simulink model "rpoco8_testbench_v11"  (Simulink 7.4)
settings: Solver=ode45  StartTime=0.0  StopTime=10.0  RelTol=1e-3
undefined symbols (not defined in the file): quant
libraries: xbsIndex_r4 x1012, xps_library x76, casper_library_misc x55, casper_library_multipliers x19, casper_library_delays x9, casper_library_sources x4, simulink x1
(part 6 of 11)

== system rpoco8_testbench_v11/xengine8_muxed/be ==
  a+bi : Inport  OutDataType=sfix(16)  OutScaling=2^0
  c+di : Inport  Port=2  OutDataType=sfix(16)  OutScaling=2^0
  new_acc : Inport  Port=3  OutDataType=sfix(16)  OutScaling=2^0
  Convert{,1..3} (4 alike) : xbsIndex_r4/Convert  arith_type="Signed  (2's comp)"  n_bits=16  bin_pt=15  quantization="Round  (unbiased: Even Values)"  overflow=Wrap  latency=0  dbl_ovrd=off  pipeline=off  block_type=convert
      settings: block_version=11.4
  Counter2 : xbsIndex_r4/Counter  cnt_type=Free Running  cnt_to=Inf  operation=Up  start_count=0  cnt_by_val=1  arith_type=Unsigned  n_bits=max(nchan+1,11)  bin_pt=0  load_pin=off  rst=on  en=on  explicit_period=on  period=1  dbl_ovrd=off  use_behavioral_HDL=off  implementation=Fabric  use_rpm=off  block_type=counter
  Delay1 : xbsIndex_r4/Delay  en=off  latency=mult_latency + add_latency  dbl_ovrd=off  reg_retiming=off  block_type=delay
  c_to_ri : casper_library_misc/c_to_ri  SystemSampleTime=-1  n_bits=n_bits  bin_pt=n_bits-1
  c_to_ri1 : casper_library_misc/c_to_ri  SystemSampleTime=-1  n_bits=n_bits  bin_pt=n_bits-1
  cmult_4bit_em* : casper_library_multipliers/cmult_4bit_em*  SystemSampleTime=-1  mult_latency=mult_latency  add_latency=add_latency
  imag : xps_library/Shared BRAM  Tag=xps:bram  SystemSampleTime=-1  arith_type="Signed  (2's comp)"  addr_width=max(nchan+1,11)  data_width=32  data_bin_pt=min(2*(n_bits-1), 30)  init_vals=0  sample_rate=1
      link override convert_din: arith_type="Signed  (2's comp)"
      link override i_poco8_128ch_v004_mod_xengine8_muxed_ab_imag_data_out: arith_type="Signed  (2's comp)"
      link override i_poco8_256ch_v000_xengine8_muxed_ab_imag_data_out: arith_type="Signed  (2's comp)"
      link override i_poco8_256ch_v004_mod_xengine8_muxed_ab_imag_data_out: arith_type="Signed  (2's comp)"
      link override i_poco8_256ch_v004_xengine8_muxed_ab_imag_data_out: arith_type="Signed  (2's comp)"
      link override i_poco8_32ch_v001_xengine8_muxed_ab_imag_data_out: arith_type="Signed  (2's comp)"
      link override i_poco8_32ch_v002_xengine8_muxed_ab_imag_data_out: arith_type="Signed  (2's comp)"
      link override i_poco8_32ch_v003_xengine8_muxed_ab_imag_data_out: arith_type="Signed  (2's comp)"
      link override i_poco8_test_xengine8_muxed_ab_imag_data_out: arith_type="Signed  (2's comp)"
      link override mem/sim_data_in: arith_type="Signed  (2's comp)"
      link override poco4_quad_200_4k_01_xengine4_x_cross_sl1_imag_data_out: arith_type="Signed  (2's comp)"
      link override r_poco8_4k_10_200_xengine8_x_cross_sl1_imag_data_out: arith_type="Signed  (2's comp)"
      link override r_poco8_4k_10_200a_xengine8_ab_imag_data_out: arith_type="Signed  (2's comp)"
  real : xps_library/Shared BRAM  Tag=xps:bram  SystemSampleTime=-1  arith_type="Signed  (2's comp)"  addr_width=max(nchan+1,11)  data_width=32  data_bin_pt=min(2*(n_bits-1), 30)  init_vals=0  sample_rate=1
      link override convert_din: arith_type="Signed  (2's comp)"
      link override i_poco8_128ch_v004_mod_xengine8_muxed_ab_real_data_out: arith_type="Signed  (2's comp)"
      link override i_poco8_256ch_v000_xengine8_muxed_ab_real_data_out: arith_type="Signed  (2's comp)"
      link override i_poco8_256ch_v004_mod_xengine8_muxed_ab_real_data_out: arith_type="Signed  (2's comp)"
      link override i_poco8_256ch_v004_xengine8_muxed_ab_real_data_out: arith_type="Signed  (2's comp)"
      link override i_poco8_32ch_v001_xengine8_muxed_ab_real_data_out: arith_type="Signed  (2's comp)"
      link override i_poco8_32ch_v002_xengine8_muxed_ab_real_data_out: arith_type="Signed  (2's comp)"
      link override i_poco8_32ch_v003_xengine8_muxed_ab_real_data_out: arith_type="Signed  (2's comp)"
      link override i_poco8_test_xengine8_muxed_ab_real_data_out: arith_type="Signed  (2's comp)"
      link override mem/sim_data_in: arith_type="Signed  (2's comp)"
      link override poco4_quad_200_4k_01_xengine4_x_cross_sl1_real_data_out: arith_type="Signed  (2's comp)"
      link override r_poco8_4k_10_200_xengine8_x_cross_sl1_real_data_out: arith_type="Signed  (2's comp)"
      link override r_poco8_4k_10_200a_xengine8_ab_real_data_out: arith_type="Signed  (2's comp)"
  simple_bram_vacc : Subsystem  AncestorBlock=casper_library/Accumulators/simple_bram_vacc  mask(simple_bram_vacc){vec_len=2048, arith_type=Signed, n_bits=32, bin_pt=30}
      mask prompts: vec_len "Vector length:"; arith_type "Output type:"; n_bits "Bit width (output):"; bin_pt "Binary point (output):"
      mask help: This simple vector accumulator is based on a shift register. It outputs its previous accumulated vector after a new_acc pulse is received.
      mask init:
      | simple_bram_vacc_init(gcb, ...
      |     'vec_len', vec_len, ...
      |     'arith_type', arith_type, ...
      |     'n_bits', n_bits, ...
      |     'bin_pt', bin_pt);
      mask StyleString: edit,popup(Signed|Unsigned),edit,edit
  simple_bram_vacc1 : Subsystem  AncestorBlock=casper_library/Accumulators/simple_bram_vacc  mask(simple_bram_vacc){vec_len=2048, arith_type=Signed, n_bits=32, bin_pt=30}
      mask prompts: vec_len "Vector length:"; arith_type "Output type:"; n_bits "Bit width (output):"; bin_pt "Binary point (output):"
      mask help: This simple vector accumulator is based on a shift register. It outputs its previous accumulated vector after a new_acc pulse is received.
      mask init:
      | simple_bram_vacc_init(gcb, ...
      |     'vec_len', vec_len, ...
      |     'arith_type', arith_type, ...
      |     'n_bits', n_bits, ...
      |     'bin_pt', bin_pt);
      mask StyleString: edit,popup(Signed|Unsigned),edit,edit
  wires:
  cmult_4bit_em*/2 -> simple_bram_vacc1/2(din)
  cmult_4bit_em*/1 -> simple_bram_vacc/2(din)
  simple_bram_vacc1/2(valid) -> imag/3
  simple_bram_vacc1/1(dout) -> imag/2
  Delay1/1 -> Counter2/1, simple_bram_vacc/1(new_acc), simple_bram_vacc1/1(new_acc)
  simple_bram_vacc/1(dout) -> real/2
  Counter2/1 -> imag/1, real/1
  simple_bram_vacc/2(valid) -> real/3, Counter2/2
  new_acc/1 -> Delay1/1
  c+di/1 -> c_to_ri1/1
  a+bi/1 -> c_to_ri/1
  c_to_ri1/2 -> Convert3/1
  c_to_ri1/1 -> Convert2/1
  c_to_ri/2 -> Convert1/1
  c_to_ri/1 -> Convert/1
  Convert/1 -> cmult_4bit_em*/1
  Convert1/1 -> cmult_4bit_em*/2
  Convert2/1 -> cmult_4bit_em*/3
  Convert3/1 -> cmult_4bit_em*/4

== system rpoco8_testbench_v11/xengine8_muxed/bf ==
  a+bi : Inport  OutDataType=sfix(16)  OutScaling=2^0
  c+di : Inport  Port=2  OutDataType=sfix(16)  OutScaling=2^0
  new_acc : Inport  Port=3  OutDataType=sfix(16)  OutScaling=2^0
  Convert{,1..3} (4 alike) : xbsIndex_r4/Convert  arith_type="Signed  (2's comp)"  n_bits=16  bin_pt=15  quantization="Round  (unbiased: Even Values)"  overflow=Wrap  latency=0  dbl_ovrd=off  pipeline=off  block_type=convert
      settings: block_version=11.4
  Counter2 : xbsIndex_r4/Counter  cnt_type=Free Running  cnt_to=Inf  operation=Up  start_count=0  cnt_by_val=1  arith_type=Unsigned  n_bits=max(nchan+1,11)  bin_pt=0  load_pin=off  rst=on  en=on  explicit_period=on  period=1  dbl_ovrd=off  use_behavioral_HDL=off  implementation=Fabric  use_rpm=off  block_type=counter
  Delay1 : xbsIndex_r4/Delay  en=off  latency=mult_latency + add_latency  dbl_ovrd=off  reg_retiming=off  block_type=delay
  c_to_ri : casper_library_misc/c_to_ri  SystemSampleTime=-1  n_bits=n_bits  bin_pt=n_bits-1
  c_to_ri1 : casper_library_misc/c_to_ri  SystemSampleTime=-1  n_bits=n_bits  bin_pt=n_bits-1
  cmult_4bit_em* : casper_library_multipliers/cmult_4bit_em*  SystemSampleTime=-1  mult_latency=mult_latency  add_latency=add_latency
  imag : xps_library/Shared BRAM  Tag=xps:bram  SystemSampleTime=-1  arith_type="Signed  (2's comp)"  addr_width=max(nchan+1,11)  data_width=32  data_bin_pt=min(2*(n_bits-1), 30)  init_vals=0  sample_rate=1
      link override convert_din: arith_type="Signed  (2's comp)"
      link override i_poco8_128ch_v004_mod_xengine8_muxed_ab_imag_data_out: arith_type="Signed  (2's comp)"
      link override i_poco8_256ch_v000_xengine8_muxed_ab_imag_data_out: arith_type="Signed  (2's comp)"
      link override i_poco8_256ch_v004_mod_xengine8_muxed_ab_imag_data_out: arith_type="Signed  (2's comp)"
      link override i_poco8_256ch_v004_xengine8_muxed_ab_imag_data_out: arith_type="Signed  (2's comp)"
      link override i_poco8_32ch_v001_xengine8_muxed_ab_imag_data_out: arith_type="Signed  (2's comp)"
      link override i_poco8_32ch_v002_xengine8_muxed_ab_imag_data_out: arith_type="Signed  (2's comp)"
      link override i_poco8_32ch_v003_xengine8_muxed_ab_imag_data_out: arith_type="Signed  (2's comp)"
      link override i_poco8_test_xengine8_muxed_ab_imag_data_out: arith_type="Signed  (2's comp)"
      link override mem/sim_data_in: arith_type="Signed  (2's comp)"
      link override poco4_quad_200_4k_01_xengine4_x_cross_sl1_imag_data_out: arith_type="Signed  (2's comp)"
      link override r_poco8_4k_10_200_xengine8_x_cross_sl1_imag_data_out: arith_type="Signed  (2's comp)"
      link override r_poco8_4k_10_200a_xengine8_ab_imag_data_out: arith_type="Signed  (2's comp)"
  real : xps_library/Shared BRAM  Tag=xps:bram  SystemSampleTime=-1  arith_type="Signed  (2's comp)"  addr_width=max(nchan+1,11)  data_width=32  data_bin_pt=min(2*(n_bits-1), 30)  init_vals=0  sample_rate=1
      link override convert_din: arith_type="Signed  (2's comp)"
      link override i_poco8_128ch_v004_mod_xengine8_muxed_ab_real_data_out: arith_type="Signed  (2's comp)"
      link override i_poco8_256ch_v000_xengine8_muxed_ab_real_data_out: arith_type="Signed  (2's comp)"
      link override i_poco8_256ch_v004_mod_xengine8_muxed_ab_real_data_out: arith_type="Signed  (2's comp)"
      link override i_poco8_256ch_v004_xengine8_muxed_ab_real_data_out: arith_type="Signed  (2's comp)"
      link override i_poco8_32ch_v001_xengine8_muxed_ab_real_data_out: arith_type="Signed  (2's comp)"
      link override i_poco8_32ch_v002_xengine8_muxed_ab_real_data_out: arith_type="Signed  (2's comp)"
      link override i_poco8_32ch_v003_xengine8_muxed_ab_real_data_out: arith_type="Signed  (2's comp)"
      link override i_poco8_test_xengine8_muxed_ab_real_data_out: arith_type="Signed  (2's comp)"
      link override mem/sim_data_in: arith_type="Signed  (2's comp)"
      link override poco4_quad_200_4k_01_xengine4_x_cross_sl1_real_data_out: arith_type="Signed  (2's comp)"
      link override r_poco8_4k_10_200_xengine8_x_cross_sl1_real_data_out: arith_type="Signed  (2's comp)"
      link override r_poco8_4k_10_200a_xengine8_ab_real_data_out: arith_type="Signed  (2's comp)"
  simple_bram_vacc : Subsystem  AncestorBlock=casper_library/Accumulators/simple_bram_vacc  mask(simple_bram_vacc){vec_len=2048, arith_type=Signed, n_bits=32, bin_pt=30}
      mask prompts: vec_len "Vector length:"; arith_type "Output type:"; n_bits "Bit width (output):"; bin_pt "Binary point (output):"
      mask help: This simple vector accumulator is based on a shift register. It outputs its previous accumulated vector after a new_acc pulse is received.
      mask init:
      | simple_bram_vacc_init(gcb, ...
      |     'vec_len', vec_len, ...
      |     'arith_type', arith_type, ...
      |     'n_bits', n_bits, ...
      |     'bin_pt', bin_pt);
      mask StyleString: edit,popup(Signed|Unsigned),edit,edit
  simple_bram_vacc1 : Subsystem  AncestorBlock=casper_library/Accumulators/simple_bram_vacc  mask(simple_bram_vacc){vec_len=2048, arith_type=Signed, n_bits=32, bin_pt=30}
      mask prompts: vec_len "Vector length:"; arith_type "Output type:"; n_bits "Bit width (output):"; bin_pt "Binary point (output):"
      mask help: This simple vector accumulator is based on a shift register. It outputs its previous accumulated vector after a new_acc pulse is received.
      mask init:
      | simple_bram_vacc_init(gcb, ...
      |     'vec_len', vec_len, ...
      |     'arith_type', arith_type, ...
      |     'n_bits', n_bits, ...
      |     'bin_pt', bin_pt);
      mask StyleString: edit,popup(Signed|Unsigned),edit,edit
  wires:
  cmult_4bit_em*/2 -> simple_bram_vacc1/2(din)
  cmult_4bit_em*/1 -> simple_bram_vacc/2(din)
  simple_bram_vacc1/2(valid) -> imag/3
  simple_bram_vacc1/1(dout) -> imag/2
  Delay1/1 -> Counter2/1, simple_bram_vacc/1(new_acc), simple_bram_vacc1/1(new_acc)
  simple_bram_vacc/1(dout) -> real/2
  Counter2/1 -> imag/1, real/1
  simple_bram_vacc/2(valid) -> real/3, Counter2/2
  new_acc/1 -> Delay1/1
  c+di/1 -> c_to_ri1/1
  a+bi/1 -> c_to_ri/1
  c_to_ri1/2 -> Convert3/1
  c_to_ri1/1 -> Convert2/1
  c_to_ri/2 -> Convert1/1
  c_to_ri/1 -> Convert/1
  Convert/1 -> cmult_4bit_em*/1
  Convert1/1 -> cmult_4bit_em*/2
  Convert2/1 -> cmult_4bit_em*/3
  Convert3/1 -> cmult_4bit_em*/4

== system rpoco8_testbench_v11/xengine8_muxed/cc ==
  a+bi : Inport  OutDataType=sfix(16)  OutScaling=2^0
  c+di : Inport  Port=2  OutDataType=sfix(16)  OutScaling=2^0
  new_acc : Inport  Port=3  OutDataType=sfix(16)  OutScaling=2^0
  Convert{,1..3} (4 alike) : xbsIndex_r4/Convert  arith_type="Signed  (2's comp)"  n_bits=16  bin_pt=15  quantization="Round  (unbiased: Even Values)"  overflow=Wrap  latency=0  dbl_ovrd=off  pipeline=off  block_type=convert
      settings: block_version=11.4
  Counter2 : xbsIndex_r4/Counter  cnt_type=Free Running  cnt_to=Inf  operation=Up  start_count=0  cnt_by_val=1  arith_type=Unsigned  n_bits=max(nchan+1,11)  bin_pt=0  load_pin=off  rst=on  en=on  explicit_period=on  period=1  dbl_ovrd=off  use_behavioral_HDL=off  implementation=Fabric  use_rpm=off  block_type=counter
  Delay1 : xbsIndex_r4/Delay  en=off  latency=mult_latency + add_latency  dbl_ovrd=off  reg_retiming=off  block_type=delay
  c_to_ri : casper_library_misc/c_to_ri  SystemSampleTime=-1  n_bits=n_bits  bin_pt=n_bits-1
  c_to_ri1 : casper_library_misc/c_to_ri  SystemSampleTime=-1  n_bits=n_bits  bin_pt=n_bits-1
  cmult_4bit_em* : casper_library_multipliers/cmult_4bit_em*  SystemSampleTime=-1  mult_latency=mult_latency  add_latency=add_latency
  real : xps_library/Shared BRAM  Tag=xps:bram  SystemSampleTime=-1  arith_type="Signed  (2's comp)"  addr_width=max(nchan+1,11)  data_width=32  data_bin_pt=min(2*(n_bits-1), 30)  init_vals=0  sample_rate=1
      link override convert_din: arith_type="Signed  (2's comp)"
      link override i_poco8_128ch_v004_mod_xengine8_muxed_aa_real_data_out: arith_type="Signed  (2's comp)"
      link override i_poco8_256ch_v000_xengine8_muxed_aa_real_data_out: arith_type="Signed  (2's comp)"
      link override i_poco8_256ch_v004_mod_xengine8_muxed_aa_real_data_out: arith_type="Signed  (2's comp)"
      link override i_poco8_256ch_v004_xengine8_muxed_aa_real_data_out: arith_type="Signed  (2's comp)"
      link override i_poco8_32ch_v001_xengine8_muxed_aa_real_data_out: arith_type="Signed  (2's comp)"
      link override i_poco8_32ch_v002_xengine8_muxed_aa_real_data_out: arith_type="Signed  (2's comp)"
      link override i_poco8_32ch_v003_xengine8_muxed_aa_real_data_out: arith_type="Signed  (2's comp)"
      link override i_poco8_test_xengine8_muxed_aa_real_data_out: arith_type="Signed  (2's comp)"
      link override mem/sim_data_in: arith_type="Signed  (2's comp)"
      link override poco4_quad_200_4k_01_xengine4_x_auto_sl_real_data_out: arith_type="Signed  (2's comp)"
      link override r_poco8_4k_10_200_xengine8_x_auto_sl_real_data_out: arith_type="Signed  (2's comp)"
      link override r_poco8_4k_10_200a_xengine8_aa_real_data_out: arith_type="Signed  (2's comp)"
  simple_bram_vacc : Subsystem  AncestorBlock=casper_library/Accumulators/simple_bram_vacc  mask(simple_bram_vacc){vec_len=2048, arith_type=Signed, n_bits=32, bin_pt=30}
      mask prompts: vec_len "Vector length:"; arith_type "Output type:"; n_bits "Bit width (output):"; bin_pt "Binary point (output):"
      mask help: This simple vector accumulator is based on a shift register. It outputs its previous accumulated vector after a new_acc pulse is received.
      mask init:
      | simple_bram_vacc_init(gcb, ...
      |     'vec_len', vec_len, ...
      |     'arith_type', arith_type, ...
      |     'n_bits', n_bits, ...
      |     'bin_pt', bin_pt);
      mask StyleString: edit,popup(Signed|Unsigned),edit,edit
  wires:
  cmult_4bit_em*/1 -> simple_bram_vacc/2(din)
  new_acc/1 -> Delay1/1
  c+di/1 -> c_to_ri1/1
  a+bi/1 -> c_to_ri/1
  simple_bram_vacc/2(valid) -> Counter2/2, real/3
  Counter2/1 -> real/1
  simple_bram_vacc/1(dout) -> real/2
  Delay1/1 -> Counter2/1, simple_bram_vacc/1(new_acc)
  c_to_ri1/2 -> Convert3/1
  c_to_ri1/1 -> Convert2/1
  c_to_ri/2 -> Convert1/1
  c_to_ri/1 -> Convert/1
  Convert/1 -> cmult_4bit_em*/1
  Convert1/1 -> cmult_4bit_em*/2
  Convert2/1 -> cmult_4bit_em*/3
  Convert3/1 -> cmult_4bit_em*/4

== system rpoco8_testbench_v11/xengine8_muxed/cd ==
  a+bi : Inport  OutDataType=sfix(16)  OutScaling=2^0
  c+di : Inport  Port=2  OutDataType=sfix(16)  OutScaling=2^0
  new_acc : Inport  Port=3  OutDataType=sfix(16)  OutScaling=2^0
  Convert{,1..3} (4 alike) : xbsIndex_r4/Convert  arith_type="Signed  (2's comp)"  n_bits=16  bin_pt=15  quantization="Round  (unbiased: Even Values)"  overflow=Wrap  latency=0  dbl_ovrd=off  pipeline=off  block_type=convert
      settings: block_version=11.4
  Counter2 : xbsIndex_r4/Counter  cnt_type=Free Running  cnt_to=Inf  operation=Up  start_count=0  cnt_by_val=1  arith_type=Unsigned  n_bits=max(nchan+1,11)  bin_pt=0  load_pin=off  rst=on  en=on  explicit_period=on  period=1  dbl_ovrd=off  use_behavioral_HDL=off  implementation=Fabric  use_rpm=off  block_type=counter
  Delay1 : xbsIndex_r4/Delay  en=off  latency=mult_latency + add_latency  dbl_ovrd=off  reg_retiming=off  block_type=delay
  c_to_ri : casper_library_misc/c_to_ri  SystemSampleTime=-1  n_bits=n_bits  bin_pt=n_bits-1
  c_to_ri1 : casper_library_misc/c_to_ri  SystemSampleTime=-1  n_bits=n_bits  bin_pt=n_bits-1
  cmult_4bit_em* : casper_library_multipliers/cmult_4bit_em*  SystemSampleTime=-1  mult_latency=mult_latency  add_latency=add_latency
  imag : xps_library/Shared BRAM  Tag=xps:bram  SystemSampleTime=-1  arith_type="Signed  (2's comp)"  addr_width=max(nchan+1,11)  data_width=32  data_bin_pt=min(2*(n_bits-1), 30)  init_vals=0  sample_rate=1
      link override convert_din: arith_type="Signed  (2's comp)"
      link override i_poco8_128ch_v004_mod_xengine8_muxed_ab_imag_data_out: arith_type="Signed  (2's comp)"
      link override i_poco8_256ch_v000_xengine8_muxed_ab_imag_data_out: arith_type="Signed  (2's comp)"
      link override i_poco8_256ch_v004_mod_xengine8_muxed_ab_imag_data_out: arith_type="Signed  (2's comp)"
      link override i_poco8_256ch_v004_xengine8_muxed_ab_imag_data_out: arith_type="Signed  (2's comp)"
      link override i_poco8_32ch_v001_xengine8_muxed_ab_imag_data_out: arith_type="Signed  (2's comp)"
      link override i_poco8_32ch_v002_xengine8_muxed_ab_imag_data_out: arith_type="Signed  (2's comp)"
      link override i_poco8_32ch_v003_xengine8_muxed_ab_imag_data_out: arith_type="Signed  (2's comp)"
      link override i_poco8_test_xengine8_muxed_ab_imag_data_out: arith_type="Signed  (2's comp)"
      link override mem/sim_data_in: arith_type="Signed  (2's comp)"
      link override poco4_quad_200_4k_01_xengine4_x_cross_sl1_imag_data_out: arith_type="Signed  (2's comp)"
      link override r_poco8_4k_10_200_xengine8_x_cross_sl1_imag_data_out: arith_type="Signed  (2's comp)"
      link override r_poco8_4k_10_200a_xengine8_ab_imag_data_out: arith_type="Signed  (2's comp)"
  real : xps_library/Shared BRAM  Tag=xps:bram  SystemSampleTime=-1  arith_type="Signed  (2's comp)"  addr_width=max(nchan+1,11)  data_width=32  data_bin_pt=min(2*(n_bits-1), 30)  init_vals=0  sample_rate=1
      link override convert_din: arith_type="Signed  (2's comp)"
      link override i_poco8_128ch_v004_mod_xengine8_muxed_ab_real_data_out: arith_type="Signed  (2's comp)"
      link override i_poco8_256ch_v000_xengine8_muxed_ab_real_data_out: arith_type="Signed  (2's comp)"
      link override i_poco8_256ch_v004_mod_xengine8_muxed_ab_real_data_out: arith_type="Signed  (2's comp)"
      link override i_poco8_256ch_v004_xengine8_muxed_ab_real_data_out: arith_type="Signed  (2's comp)"
      link override i_poco8_32ch_v001_xengine8_muxed_ab_real_data_out: arith_type="Signed  (2's comp)"
      link override i_poco8_32ch_v002_xengine8_muxed_ab_real_data_out: arith_type="Signed  (2's comp)"
      link override i_poco8_32ch_v003_xengine8_muxed_ab_real_data_out: arith_type="Signed  (2's comp)"
      link override i_poco8_test_xengine8_muxed_ab_real_data_out: arith_type="Signed  (2's comp)"
      link override mem/sim_data_in: arith_type="Signed  (2's comp)"
      link override poco4_quad_200_4k_01_xengine4_x_cross_sl1_real_data_out: arith_type="Signed  (2's comp)"
      link override r_poco8_4k_10_200_xengine8_x_cross_sl1_real_data_out: arith_type="Signed  (2's comp)"
      link override r_poco8_4k_10_200a_xengine8_ab_real_data_out: arith_type="Signed  (2's comp)"
  simple_bram_vacc : Subsystem  AncestorBlock=casper_library/Accumulators/simple_bram_vacc  mask(simple_bram_vacc){vec_len=2048, arith_type=Signed, n_bits=32, bin_pt=30}
      mask prompts: vec_len "Vector length:"; arith_type "Output type:"; n_bits "Bit width (output):"; bin_pt "Binary point (output):"
      mask help: This simple vector accumulator is based on a shift register. It outputs its previous accumulated vector after a new_acc pulse is received.
      mask init:
      | simple_bram_vacc_init(gcb, ...
      |     'vec_len', vec_len, ...
      |     'arith_type', arith_type, ...
      |     'n_bits', n_bits, ...
      |     'bin_pt', bin_pt);
      mask StyleString: edit,popup(Signed|Unsigned),edit,edit
  simple_bram_vacc1 : Subsystem  AncestorBlock=casper_library/Accumulators/simple_bram_vacc  mask(simple_bram_vacc){vec_len=2048, arith_type=Signed, n_bits=32, bin_pt=30}
      mask prompts: vec_len "Vector length:"; arith_type "Output type:"; n_bits "Bit width (output):"; bin_pt "Binary point (output):"
      mask help: This simple vector accumulator is based on a shift register. It outputs its previous accumulated vector after a new_acc pulse is received.
      mask init:
      | simple_bram_vacc_init(gcb, ...
      |     'vec_len', vec_len, ...
      |     'arith_type', arith_type, ...
      |     'n_bits', n_bits, ...
      |     'bin_pt', bin_pt);
      mask StyleString: edit,popup(Signed|Unsigned),edit,edit
  wires:
  cmult_4bit_em*/2 -> simple_bram_vacc1/2(din)
  cmult_4bit_em*/1 -> simple_bram_vacc/2(din)
  simple_bram_vacc1/2(valid) -> imag/3
  simple_bram_vacc1/1(dout) -> imag/2
  Delay1/1 -> Counter2/1, simple_bram_vacc/1(new_acc), simple_bram_vacc1/1(new_acc)
  simple_bram_vacc/1(dout) -> real/2
  Counter2/1 -> imag/1, real/1
  simple_bram_vacc/2(valid) -> real/3, Counter2/2
  new_acc/1 -> Delay1/1
  c+di/1 -> c_to_ri1/1
  a+bi/1 -> c_to_ri/1
  c_to_ri1/2 -> Convert3/1
  c_to_ri1/1 -> Convert2/1
  c_to_ri/2 -> Convert1/1
  c_to_ri/1 -> Convert/1
  Convert/1 -> cmult_4bit_em*/1
  Convert1/1 -> cmult_4bit_em*/2
  Convert2/1 -> cmult_4bit_em*/3
  Convert3/1 -> cmult_4bit_em*/4

== system rpoco8_testbench_v11/xengine8_muxed/cg ==
  a+bi : Inport  OutDataType=sfix(16)  OutScaling=2^0
  c+di : Inport  Port=2  OutDataType=sfix(16)  OutScaling=2^0
  new_acc : Inport  Port=3  OutDataType=sfix(16)  OutScaling=2^0
  Convert{,1..3} (4 alike) : xbsIndex_r4/Convert  arith_type="Signed  (2's comp)"  n_bits=16  bin_pt=15  quantization="Round  (unbiased: Even Values)"  overflow=Wrap  latency=0  dbl_ovrd=off  pipeline=off  block_type=convert
      settings: block_version=11.4
  Counter2 : xbsIndex_r4/Counter  cnt_type=Free Running  cnt_to=Inf  operation=Up  start_count=0  cnt_by_val=1  arith_type=Unsigned  n_bits=max(nchan+1,11)  bin_pt=0  load_pin=off  rst=on  en=on  explicit_period=on  period=1  dbl_ovrd=off  use_behavioral_HDL=off  implementation=Fabric  use_rpm=off  block_type=counter
  Delay1 : xbsIndex_r4/Delay  en=off  latency=mult_latency + add_latency  dbl_ovrd=off  reg_retiming=off  block_type=delay
  c_to_ri : casper_library_misc/c_to_ri  SystemSampleTime=-1  n_bits=n_bits  bin_pt=n_bits-1
  c_to_ri1 : casper_library_misc/c_to_ri  SystemSampleTime=-1  n_bits=n_bits  bin_pt=n_bits-1
  cmult_4bit_em* : casper_library_multipliers/cmult_4bit_em*  SystemSampleTime=-1  mult_latency=mult_latency  add_latency=add_latency
  imag : xps_library/Shared BRAM  Tag=xps:bram  SystemSampleTime=-1  arith_type="Signed  (2's comp)"  addr_width=max(nchan+1,11)  data_width=32  data_bin_pt=min(2*(n_bits-1), 30)  init_vals=0  sample_rate=1
      link override convert_din: arith_type="Signed  (2's comp)"
      link override i_poco8_128ch_v004_mod_xengine8_muxed_ab_imag_data_out: arith_type="Signed  (2's comp)"
      link override i_poco8_256ch_v000_xengine8_muxed_ab_imag_data_out: arith_type="Signed  (2's comp)"
      link override i_poco8_256ch_v004_mod_xengine8_muxed_ab_imag_data_out: arith_type="Signed  (2's comp)"
      link override i_poco8_256ch_v004_xengine8_muxed_ab_imag_data_out: arith_type="Signed  (2's comp)"
      link override i_poco8_32ch_v001_xengine8_muxed_ab_imag_data_out: arith_type="Signed  (2's comp)"
      link override i_poco8_32ch_v002_xengine8_muxed_ab_imag_data_out: arith_type="Signed  (2's comp)"
      link override i_poco8_32ch_v003_xengine8_muxed_ab_imag_data_out: arith_type="Signed  (2's comp)"
      link override i_poco8_test_xengine8_muxed_ab_imag_data_out: arith_type="Signed  (2's comp)"
      link override mem/sim_data_in: arith_type="Signed  (2's comp)"
      link override poco4_quad_200_4k_01_xengine4_x_cross_sl1_imag_data_out: arith_type="Signed  (2's comp)"
      link override r_poco8_4k_10_200_xengine8_x_cross_sl1_imag_data_out: arith_type="Signed  (2's comp)"
      link override r_poco8_4k_10_200a_xengine8_ab_imag_data_out: arith_type="Signed  (2's comp)"
  real : xps_library/Shared BRAM  Tag=xps:bram  SystemSampleTime=-1  arith_type="Signed  (2's comp)"  addr_width=max(nchan+1,11)  data_width=32  data_bin_pt=min(2*(n_bits-1), 30)  init_vals=0  sample_rate=1
      link override convert_din: arith_type="Signed  (2's comp)"
      link override i_poco8_128ch_v004_mod_xengine8_muxed_ab_real_data_out: arith_type="Signed  (2's comp)"
      link override i_poco8_256ch_v000_xengine8_muxed_ab_real_data_out: arith_type="Signed  (2's comp)"
      link override i_poco8_256ch_v004_mod_xengine8_muxed_ab_real_data_out: arith_type="Signed  (2's comp)"
      link override i_poco8_256ch_v004_xengine8_muxed_ab_real_data_out: arith_type="Signed  (2's comp)"
      link override i_poco8_32ch_v001_xengine8_muxed_ab_real_data_out: arith_type="Signed  (2's comp)"
      link override i_poco8_32ch_v002_xengine8_muxed_ab_real_data_out: arith_type="Signed  (2's comp)"
      link override i_poco8_32ch_v003_xengine8_muxed_ab_real_data_out: arith_type="Signed  (2's comp)"
      link override i_poco8_test_xengine8_muxed_ab_real_data_out: arith_type="Signed  (2's comp)"
      link override mem/sim_data_in: arith_type="Signed  (2's comp)"
      link override poco4_quad_200_4k_01_xengine4_x_cross_sl1_real_data_out: arith_type="Signed  (2's comp)"
      link override r_poco8_4k_10_200_xengine8_x_cross_sl1_real_data_out: arith_type="Signed  (2's comp)"
      link override r_poco8_4k_10_200a_xengine8_ab_real_data_out: arith_type="Signed  (2's comp)"
  simple_bram_vacc : Subsystem  AncestorBlock=casper_library/Accumulators/simple_bram_vacc  mask(simple_bram_vacc){vec_len=2048, arith_type=Signed, n_bits=32, bin_pt=30}
      mask prompts: vec_len "Vector length:"; arith_type "Output type:"; n_bits "Bit width (output):"; bin_pt "Binary point (output):"
      mask help: This simple vector accumulator is based on a shift register. It outputs its previous accumulated vector after a new_acc pulse is received.
      mask init:
      | simple_bram_vacc_init(gcb, ...
      |     'vec_len', vec_len, ...
      |     'arith_type', arith_type, ...
      |     'n_bits', n_bits, ...
      |     'bin_pt', bin_pt);
      mask StyleString: edit,popup(Signed|Unsigned),edit,edit
  simple_bram_vacc1 : Subsystem  AncestorBlock=casper_library/Accumulators/simple_bram_vacc  mask(simple_bram_vacc){vec_len=2048, arith_type=Signed, n_bits=32, bin_pt=30}
      mask prompts: vec_len "Vector length:"; arith_type "Output type:"; n_bits "Bit width (output):"; bin_pt "Binary point (output):"
      mask help: This simple vector accumulator is based on a shift register. It outputs its previous accumulated vector after a new_acc pulse is received.
      mask init:
      | simple_bram_vacc_init(gcb, ...
      |     'vec_len', vec_len, ...
      |     'arith_type', arith_type, ...
      |     'n_bits', n_bits, ...
      |     'bin_pt', bin_pt);
      mask StyleString: edit,popup(Signed|Unsigned),edit,edit
  wires:
  cmult_4bit_em*/2 -> simple_bram_vacc1/2(din)
  cmult_4bit_em*/1 -> simple_bram_vacc/2(din)
  simple_bram_vacc1/2(valid) -> imag/3
  simple_bram_vacc1/1(dout) -> imag/2
  Delay1/1 -> Counter2/1, simple_bram_vacc/1(new_acc), simple_bram_vacc1/1(new_acc)
  simple_bram_vacc/1(dout) -> real/2
  Counter2/1 -> imag/1, real/1
  simple_bram_vacc/2(valid) -> real/3, Counter2/2
  new_acc/1 -> Delay1/1
  c+di/1 -> c_to_ri1/1
  a+bi/1 -> c_to_ri/1
  c_to_ri1/2 -> Convert3/1
  c_to_ri1/1 -> Convert2/1
  c_to_ri/2 -> Convert1/1
  c_to_ri/1 -> Convert/1
  Convert/1 -> cmult_4bit_em*/1
  Convert1/1 -> cmult_4bit_em*/2
  Convert2/1 -> cmult_4bit_em*/3
  Convert3/1 -> cmult_4bit_em*/4

== system rpoco8_testbench_v11/xengine8_muxed/ch ==
  a+bi : Inport  OutDataType=sfix(16)  OutScaling=2^0
  c+di : Inport  Port=2  OutDataType=sfix(16)  OutScaling=2^0
  new_acc : Inport  Port=3  OutDataType=sfix(16)  OutScaling=2^0
  Convert{,1..3} (4 alike) : xbsIndex_r4/Convert  arith_type="Signed  (2's comp)"  n_bits=16  bin_pt=15  quantization="Round  (unbiased: Even Values)"  overflow=Wrap  latency=0  dbl_ovrd=off  pipeline=off  block_type=convert
      settings: block_version=11.4
  Counter2 : xbsIndex_r4/Counter  cnt_type=Free Running  cnt_to=Inf  operation=Up  start_count=0  cnt_by_val=1  arith_type=Unsigned  n_bits=max(nchan+1,11)  bin_pt=0  load_pin=off  rst=on  en=on  explicit_period=on  period=1  dbl_ovrd=off  use_behavioral_HDL=off  implementation=Fabric  use_rpm=off  block_type=counter
  Delay1 : xbsIndex_r4/Delay  en=off  latency=mult_latency + add_latency  dbl_ovrd=off  reg_retiming=off  block_type=delay
  c_to_ri : casper_library_misc/c_to_ri  SystemSampleTime=-1  n_bits=n_bits  bin_pt=n_bits-1
  c_to_ri1 : casper_library_misc/c_to_ri  SystemSampleTime=-1  n_bits=n_bits  bin_pt=n_bits-1
  cmult_4bit_em* : casper_library_multipliers/cmult_4bit_em*  SystemSampleTime=-1  mult_latency=mult_latency  add_latency=add_latency
  imag : xps_library/Shared BRAM  Tag=xps:bram  SystemSampleTime=-1  arith_type="Signed  (2's comp)"  addr_width=max(nchan+1,11)  data_width=32  data_bin_pt=min(2*(n_bits-1), 30)  init_vals=0  sample_rate=1
      link override convert_din: arith_type="Signed  (2's comp)"
      link override i_poco8_128ch_v004_mod_xengine8_muxed_ab_imag_data_out: arith_type="Signed  (2's comp)"
      link override i_poco8_256ch_v000_xengine8_muxed_ab_imag_data_out: arith_type="Signed  (2's comp)"
      link override i_poco8_256ch_v004_mod_xengine8_muxed_ab_imag_data_out: arith_type="Signed  (2's comp)"
      link override i_poco8_256ch_v004_xengine8_muxed_ab_imag_data_out: arith_type="Signed  (2's comp)"
      link override i_poco8_32ch_v001_xengine8_muxed_ab_imag_data_out: arith_type="Signed  (2's comp)"
      link override i_poco8_32ch_v002_xengine8_muxed_ab_imag_data_out: arith_type="Signed  (2's comp)"
      link override i_poco8_32ch_v003_xengine8_muxed_ab_imag_data_out: arith_type="Signed  (2's comp)"
      link override i_poco8_test_xengine8_muxed_ab_imag_data_out: arith_type="Signed  (2's comp)"
      link override mem/sim_data_in: arith_type="Signed  (2's comp)"
      link override poco4_quad_200_4k_01_xengine4_x_cross_sl1_imag_data_out: arith_type="Signed  (2's comp)"
      link override r_poco8_4k_10_200_xengine8_x_cross_sl1_imag_data_out: arith_type="Signed  (2's comp)"
      link override r_poco8_4k_10_200a_xengine8_ab_imag_data_out: arith_type="Signed  (2's comp)"
  real : xps_library/Shared BRAM  Tag=xps:bram  SystemSampleTime=-1  arith_type="Signed  (2's comp)"  addr_width=max(nchan+1,11)  data_width=32  data_bin_pt=min(2*(n_bits-1), 30)  init_vals=0  sample_rate=1
      link override convert_din: arith_type="Signed  (2's comp)"
      link override i_poco8_128ch_v004_mod_xengine8_muxed_ab_real_data_out: arith_type="Signed  (2's comp)"
      link override i_poco8_256ch_v000_xengine8_muxed_ab_real_data_out: arith_type="Signed  (2's comp)"
      link override i_poco8_256ch_v004_mod_xengine8_muxed_ab_real_data_out: arith_type="Signed  (2's comp)"
      link override i_poco8_256ch_v004_xengine8_muxed_ab_real_data_out: arith_type="Signed  (2's comp)"
      link override i_poco8_32ch_v001_xengine8_muxed_ab_real_data_out: arith_type="Signed  (2's comp)"
      link override i_poco8_32ch_v002_xengine8_muxed_ab_real_data_out: arith_type="Signed  (2's comp)"
      link override i_poco8_32ch_v003_xengine8_muxed_ab_real_data_out: arith_type="Signed  (2's comp)"
      link override i_poco8_test_xengine8_muxed_ab_real_data_out: arith_type="Signed  (2's comp)"
      link override mem/sim_data_in: arith_type="Signed  (2's comp)"
      link override poco4_quad_200_4k_01_xengine4_x_cross_sl1_real_data_out: arith_type="Signed  (2's comp)"
      link override r_poco8_4k_10_200_xengine8_x_cross_sl1_real_data_out: arith_type="Signed  (2's comp)"
      link override r_poco8_4k_10_200a_xengine8_ab_real_data_out: arith_type="Signed  (2's comp)"
  simple_bram_vacc : Subsystem  AncestorBlock=casper_library/Accumulators/simple_bram_vacc  mask(simple_bram_vacc){vec_len=2048, arith_type=Signed, n_bits=32, bin_pt=30}
      mask prompts: vec_len "Vector length:"; arith_type "Output type:"; n_bits "Bit width (output):"; bin_pt "Binary point (output):"
      mask help: This simple vector accumulator is based on a shift register. It outputs its previous accumulated vector after a new_acc pulse is received.
      mask init:
      | simple_bram_vacc_init(gcb, ...
      |     'vec_len', vec_len, ...
      |     'arith_type', arith_type, ...
      |     'n_bits', n_bits, ...
      |     'bin_pt', bin_pt);
      mask StyleString: edit,popup(Signed|Unsigned),edit,edit
  simple_bram_vacc1 : Subsystem  AncestorBlock=casper_library/Accumulators/simple_bram_vacc  mask(simple_bram_vacc){vec_len=2048, arith_type=Signed, n_bits=32, bin_pt=30}
      mask prompts: vec_len "Vector length:"; arith_type "Output type:"; n_bits "Bit width (output):"; bin_pt "Binary point (output):"
      mask help: This simple vector accumulator is based on a shift register. It outputs its previous accumulated vector after a new_acc pulse is received.
      mask init:
      | simple_bram_vacc_init(gcb, ...
      |     'vec_len', vec_len, ...
      |     'arith_type', arith_type, ...
      |     'n_bits', n_bits, ...
      |     'bin_pt', bin_pt);
      mask StyleString: edit,popup(Signed|Unsigned),edit,edit
  wires:
  cmult_4bit_em*/2 -> simple_bram_vacc1/2(din)
  cmult_4bit_em*/1 -> simple_bram_vacc/2(din)
  simple_bram_vacc1/2(valid) -> imag/3
  simple_bram_vacc1/1(dout) -> imag/2
  Delay1/1 -> Counter2/1, simple_bram_vacc/1(new_acc), simple_bram_vacc1/1(new_acc)
  simple_bram_vacc/1(dout) -> real/2
  Counter2/1 -> imag/1, real/1
  simple_bram_vacc/2(valid) -> real/3, Counter2/2
  new_acc/1 -> Delay1/1
  c+di/1 -> c_to_ri1/1
  a+bi/1 -> c_to_ri/1
  c_to_ri1/2 -> Convert3/1
  c_to_ri1/1 -> Convert2/1
  c_to_ri/2 -> Convert1/1
  c_to_ri/1 -> Convert/1
  Convert/1 -> cmult_4bit_em*/1
  Convert1/1 -> cmult_4bit_em*/2
  Convert2/1 -> cmult_4bit_em*/3
  Convert3/1 -> cmult_4bit_em*/4

== system rpoco8_testbench_v11/xengine8_muxed/dd ==
  a+bi : Inport  OutDataType=sfix(16)  OutScaling=2^0
  c+di : Inport  Port=2  OutDataType=sfix(16)  OutScaling=2^0
  new_acc : Inport  Port=3  OutDataType=sfix(16)  OutScaling=2^0
  Convert{,1..3} (4 alike) : xbsIndex_r4/Convert  arith_type="Signed  (2's comp)"  n_bits=16  bin_pt=15  quantization="Round  (unbiased: Even Values)"  overflow=Wrap  latency=0  dbl_ovrd=off  pipeline=off  block_type=convert
      settings: block_version=11.4
  Counter2 : xbsIndex_r4/Counter  cnt_type=Free Running  cnt_to=Inf  operation=Up  start_count=0  cnt_by_val=1  arith_type=Unsigned  n_bits=max(nchan+1,11)  bin_pt=0  load_pin=off  rst=on  en=on  explicit_period=on  period=1  dbl_ovrd=off  use_behavioral_HDL=off  implementation=Fabric  use_rpm=off  block_type=counter
  Delay1 : xbsIndex_r4/Delay  en=off  latency=mult_latency + add_latency  dbl_ovrd=off  reg_retiming=off  block_type=delay
  c_to_ri : casper_library_misc/c_to_ri  SystemSampleTime=-1  n_bits=n_bits  bin_pt=n_bits-1
  c_to_ri1 : casper_library_misc/c_to_ri  SystemSampleTime=-1  n_bits=n_bits  bin_pt=n_bits-1
  cmult_4bit_em* : casper_library_multipliers/cmult_4bit_em*  SystemSampleTime=-1  mult_latency=mult_latency  add_latency=add_latency
  real : xps_library/Shared BRAM  Tag=xps:bram  SystemSampleTime=-1  arith_type="Signed  (2's comp)"  addr_width=max(nchan+1,11)  data_width=32  data_bin_pt=min(2*(n_bits-1), 30)  init_vals=0  sample_rate=1
      link override convert_din: arith_type="Signed  (2's comp)"
      link override i_poco8_128ch_v004_mod_xengine8_muxed_aa_real_data_out: arith_type="Signed  (2's comp)"
      link override i_poco8_256ch_v000_xengine8_muxed_aa_real_data_out: arith_type="Signed  (2's comp)"
      link override i_poco8_256ch_v004_mod_xengine8_muxed_aa_real_data_out: arith_type="Signed  (2's comp)"
      link override i_poco8_256ch_v004_xengine8_muxed_aa_real_data_out: arith_type="Signed  (2's comp)"
      link override i_poco8_32ch_v001_xengine8_muxed_aa_real_data_out: arith_type="Signed  (2's comp)"
      link override i_poco8_32ch_v002_xengine8_muxed_aa_real_data_out: arith_type="Signed  (2's comp)"
      link override i_poco8_32ch_v003_xengine8_muxed_aa_real_data_out: arith_type="Signed  (2's comp)"
      link override i_poco8_test_xengine8_muxed_aa_real_data_out: arith_type="Signed  (2's comp)"
      link override mem/sim_data_in: arith_type="Signed  (2's comp)"
      link override poco4_quad_200_4k_01_xengine4_x_auto_sl_real_data_out: arith_type="Signed  (2's comp)"
      link override r_poco8_4k_10_200_xengine8_x_auto_sl_real_data_out: arith_type="Signed  (2's comp)"
      link override r_poco8_4k_10_200a_xengine8_aa_real_data_out: arith_type="Signed  (2's comp)"
  simple_bram_vacc : Subsystem  AncestorBlock=casper_library/Accumulators/simple_bram_vacc  mask(simple_bram_vacc){vec_len=2048, arith_type=Signed, n_bits=32, bin_pt=30}
      mask prompts: vec_len "Vector length:"; arith_type "Output type:"; n_bits "Bit width (output):"; bin_pt "Binary point (output):"
      mask help: This simple vector accumulator is based on a shift register. It outputs its previous accumulated vector after a new_acc pulse is received.
      mask init:
      | simple_bram_vacc_init(gcb, ...
      |     'vec_len', vec_len, ...
      |     'arith_type', arith_type, ...
      |     'n_bits', n_bits, ...
      |     'bin_pt', bin_pt);
      mask StyleString: edit,popup(Signed|Unsigned),edit,edit
  wires:
  cmult_4bit_em*/1 -> simple_bram_vacc/2(din)
  new_acc/1 -> Delay1/1
  c+di/1 -> c_to_ri1/1
  a+bi/1 -> c_to_ri/1
  simple_bram_vacc/2(valid) -> Counter2/2, real/3
  Counter2/1 -> real/1
  simple_bram_vacc/1(dout) -> real/2
  Delay1/1 -> Counter2/1, simple_bram_vacc/1(new_acc)
  c_to_ri1/2 -> Convert3/1
  c_to_ri1/1 -> Convert2/1
  c_to_ri/2 -> Convert1/1
  c_to_ri/1 -> Convert/1
  Convert/1 -> cmult_4bit_em*/1
  Convert1/1 -> cmult_4bit_em*/2
  Convert2/1 -> cmult_4bit_em*/3
  Convert3/1 -> cmult_4bit_em*/4

== system rpoco8_testbench_v11/xengine8_muxed/dg ==
  a+bi : Inport  OutDataType=sfix(16)  OutScaling=2^0
  c+di : Inport  Port=2  OutDataType=sfix(16)  OutScaling=2^0
  new_acc : Inport  Port=3  OutDataType=sfix(16)  OutScaling=2^0
  Convert{,1..3} (4 alike) : xbsIndex_r4/Convert  arith_type="Signed  (2's comp)"  n_bits=16  bin_pt=15  quantization="Round  (unbiased: Even Values)"  overflow=Wrap  latency=0  dbl_ovrd=off  pipeline=off  block_type=convert
      settings: block_version=11.4
  Counter2 : xbsIndex_r4/Counter  cnt_type=Free Running  cnt_to=Inf  operation=Up  start_count=0  cnt_by_val=1  arith_type=Unsigned  n_bits=max(nchan+1,11)  bin_pt=0  load_pin=off  rst=on  en=on  explicit_period=on  period=1  dbl_ovrd=off  use_behavioral_HDL=off  implementation=Fabric  use_rpm=off  block_type=counter
  Delay1 : xbsIndex_r4/Delay  en=off  latency=mult_latency + add_latency  dbl_ovrd=off  reg_retiming=off  block_type=delay
  c_to_ri : casper_library_misc/c_to_ri  SystemSampleTime=-1  n_bits=n_bits  bin_pt=n_bits-1
  c_to_ri1 : casper_library_misc/c_to_ri  SystemSampleTime=-1  n_bits=n_bits  bin_pt=n_bits-1
  cmult_4bit_em* : casper_library_multipliers/cmult_4bit_em*  SystemSampleTime=-1  mult_latency=mult_latency  add_latency=add_latency
  imag : xps_library/Shared BRAM  Tag=xps:bram  SystemSampleTime=-1  arith_type="Signed  (2's comp)"  addr_width=max(nchan+1,11)  data_width=32  data_bin_pt=min(2*(n_bits-1), 30)  init_vals=0  sample_rate=1
      link override convert_din: arith_type="Signed  (2's comp)"
      link override i_poco8_128ch_v004_mod_xengine8_muxed_ab_imag_data_out: arith_type="Signed  (2's comp)"
      link override i_poco8_256ch_v000_xengine8_muxed_ab_imag_data_out: arith_type="Signed  (2's comp)"
      link override i_poco8_256ch_v004_mod_xengine8_muxed_ab_imag_data_out: arith_type="Signed  (2's comp)"
      link override i_poco8_256ch_v004_xengine8_muxed_ab_imag_data_out: arith_type="Signed  (2's comp)"
      link override i_poco8_32ch_v001_xengine8_muxed_ab_imag_data_out: arith_type="Signed  (2's comp)"
      link override i_poco8_32ch_v002_xengine8_muxed_ab_imag_data_out: arith_type="Signed  (2's comp)"
      link override i_poco8_32ch_v003_xengine8_muxed_ab_imag_data_out: arith_type="Signed  (2's comp)"
      link override i_poco8_test_xengine8_muxed_ab_imag_data_out: arith_type="Signed  (2's comp)"
      link override mem/sim_data_in: arith_type="Signed  (2's comp)"
      link override poco4_quad_200_4k_01_xengine4_x_cross_sl1_imag_data_out: arith_type="Signed  (2's comp)"
      link override r_poco8_4k_10_200_xengine8_x_cross_sl1_imag_data_out: arith_type="Signed  (2's comp)"
      link override r_poco8_4k_10_200a_xengine8_ab_imag_data_out: arith_type="Signed  (2's comp)"
  real : xps_library/Shared BRAM  Tag=xps:bram  SystemSampleTime=-1  arith_type="Signed  (2's comp)"  addr_width=max(nchan+1,11)  data_width=32  data_bin_pt=min(2*(n_bits-1), 30)  init_vals=0  sample_rate=1
      link override convert_din: arith_type="Signed  (2's comp)"
      link override i_poco8_128ch_v004_mod_xengine8_muxed_ab_real_data_out: arith_type="Signed  (2's comp)"
      link override i_poco8_256ch_v000_xengine8_muxed_ab_real_data_out: arith_type="Signed  (2's comp)"
      link override i_poco8_256ch_v004_mod_xengine8_muxed_ab_real_data_out: arith_type="Signed  (2's comp)"
      link override i_poco8_256ch_v004_xengine8_muxed_ab_real_data_out: arith_type="Signed  (2's comp)"
      link override i_poco8_32ch_v001_xengine8_muxed_ab_real_data_out: arith_type="Signed  (2's comp)"
      link override i_poco8_32ch_v002_xengine8_muxed_ab_real_data_out: arith_type="Signed  (2's comp)"
      link override i_poco8_32ch_v003_xengine8_muxed_ab_real_data_out: arith_type="Signed  (2's comp)"
      link override i_poco8_test_xengine8_muxed_ab_real_data_out: arith_type="Signed  (2's comp)"
      link override mem/sim_data_in: arith_type="Signed  (2's comp)"
      link override poco4_quad_200_4k_01_xengine4_x_cross_sl1_real_data_out: arith_type="Signed  (2's comp)"
      link override r_poco8_4k_10_200_xengine8_x_cross_sl1_real_data_out: arith_type="Signed  (2's comp)"
      link override r_poco8_4k_10_200a_xengine8_ab_real_data_out: arith_type="Signed  (2's comp)"
  simple_bram_vacc : Subsystem  AncestorBlock=casper_library/Accumulators/simple_bram_vacc  mask(simple_bram_vacc){vec_len=2048, arith_type=Signed, n_bits=32, bin_pt=30}
      mask prompts: vec_len "Vector length:"; arith_type "Output type:"; n_bits "Bit width (output):"; bin_pt "Binary point (output):"
      mask help: This simple vector accumulator is based on a shift register. It outputs its previous accumulated vector after a new_acc pulse is received.
      mask init:
      | simple_bram_vacc_init(gcb, ...
      |     'vec_len', vec_len, ...
      |     'arith_type', arith_type, ...
      |     'n_bits', n_bits, ...
      |     'bin_pt', bin_pt);
      mask StyleString: edit,popup(Signed|Unsigned),edit,edit
  simple_bram_vacc1 : Subsystem  AncestorBlock=casper_library/Accumulators/simple_bram_vacc  mask(simple_bram_vacc){vec_len=2048, arith_type=Signed, n_bits=32, bin_pt=30}
      mask prompts: vec_len "Vector length:"; arith_type "Output type:"; n_bits "Bit width (output):"; bin_pt "Binary point (output):"
      mask help: This simple vector accumulator is based on a shift register. It outputs its previous accumulated vector after a new_acc pulse is received.
      mask init:
      | simple_bram_vacc_init(gcb, ...
      |     'vec_len', vec_len, ...
      |     'arith_type', arith_type, ...
      |     'n_bits', n_bits, ...
      |     'bin_pt', bin_pt);
      mask StyleString: edit,popup(Signed|Unsigned),edit,edit
  wires:
  cmult_4bit_em*/2 -> simple_bram_vacc1/2(din)
  cmult_4bit_em*/1 -> simple_bram_vacc/2(din)
  simple_bram_vacc1/2(valid) -> imag/3
  simple_bram_vacc1/1(dout) -> imag/2
  Delay1/1 -> Counter2/1, simple_bram_vacc/1(new_acc), simple_bram_vacc1/1(new_acc)
  simple_bram_vacc/1(dout) -> real/2
  Counter2/1 -> imag/1, real/1
  simple_bram_vacc/2(valid) -> real/3, Counter2/2
  new_acc/1 -> Delay1/1
  c+di/1 -> c_to_ri1/1
  a+bi/1 -> c_to_ri/1
  c_to_ri1/2 -> Convert3/1
  c_to_ri1/1 -> Convert2/1
  c_to_ri/2 -> Convert1/1
  c_to_ri/1 -> Convert/1
  Convert/1 -> cmult_4bit_em*/1
  Convert1/1 -> cmult_4bit_em*/2
  Convert2/1 -> cmult_4bit_em*/3
  Convert3/1 -> cmult_4bit_em*/4
